simulink model "HvacAirTEstimdAvg"  (R2022b)
settings: Solver=FixedStepDiscrete  StartTime=0.0  StopTime=10.0  FixedStep=0.2  RelTol=1e-3
settings Model: Creator=blue.ge  LastModifiedBy=blue.ge
settings DataIOCC: SaveFormat=StructureWithTime
settings OptimizationCC: DefaultParameterBehavior=Inlined  EnhancedBackFolding=on  PassReuseOutputArgsAs=Individual arguments  ZeroExternalMemoryAtStartup=off  ZeroInternalMemoryAtStartup=off  OptimizeBlockOrder=speed  DifferentSizesBufferReuse=on  OptimizationCustomize=off  EfficientTunableParamExpr=on
settings DebuggingCC: ParameterTunabilityLossMsg=error
settings ModelReferenceCC: ModelReferenceNumInstancesAllowed=Single
settings SFSimCC: MATLABDynamicMemAlloc=off
settings RTWCC: Description=AUTOSAR  SystemTargetFile=autosar.tlc  GenCodeOnly=on  TemplateMakefile=ert_default_tmf
settings CodeAppCC: ForceParamTrailComments=on  IgnoreCustomStorageClasses=off  OperatorAnnotations=on  SimulinkDataObjDesc=on  SFDataObjDesc=on  InsertBlockDesc=on
settings STFCustomTargetCC: TargetLangStandard=C99 (ISO)  MultiwordLength=256  ParMdlRefBuildCompliant=on  CompOptLevelCompliant=on  IncludeMdlTerminateFcn=off  CombineOutputUpdateFcns=on  SuppressErrorStatus=on  ERTCustomFileBanners=on  SupportNonFinite=off  SupportComplex=off  SupportContinuousTime=off  SupportNonInlinedSFcns=off  RemoveResetFunc=on  MATLABClassNameForMDSCustomization=Simulink.SoftwareTarget.ERTCustomization  AutosarCompliant=on  UseToolchainInfoCompliant=on  LookupTableObjectStructAxisOrder=2,1,3,4,...  LUTObjectStructOrderEvenSpacing=Size,Table,Breakpoints  SystemTargetFile=autosar.tlc
settings ERTTargetCC: TargetLangStandard=C99 (ISO)  ConcurrentExecutionCompliant=off  IncludeMdlTerminateFcn=off  SuppressErrorStatus=on  SupportNonFinite=off  SupportComplex=off  ModelStepFunctionPrototypeControlCompliant=off  CPPClassGenCompliant=off  AutosarCompliant=on  LookupTableObjectStructAxisOrder=2,1,3,4,...  LUTObjectStructOrderEvenSpacing=Size,Table,Breakpoints  InlinedParameterPlacement=NonHierarchical  ERTCustomFileTemplate=example_file_process.tlc

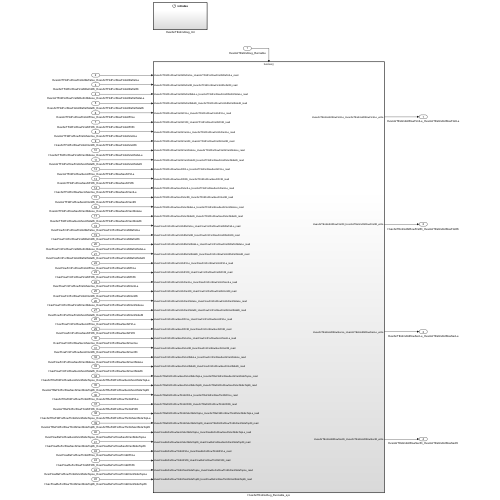
[thumbnail: Simulink's stored preview of the top-level diagram]
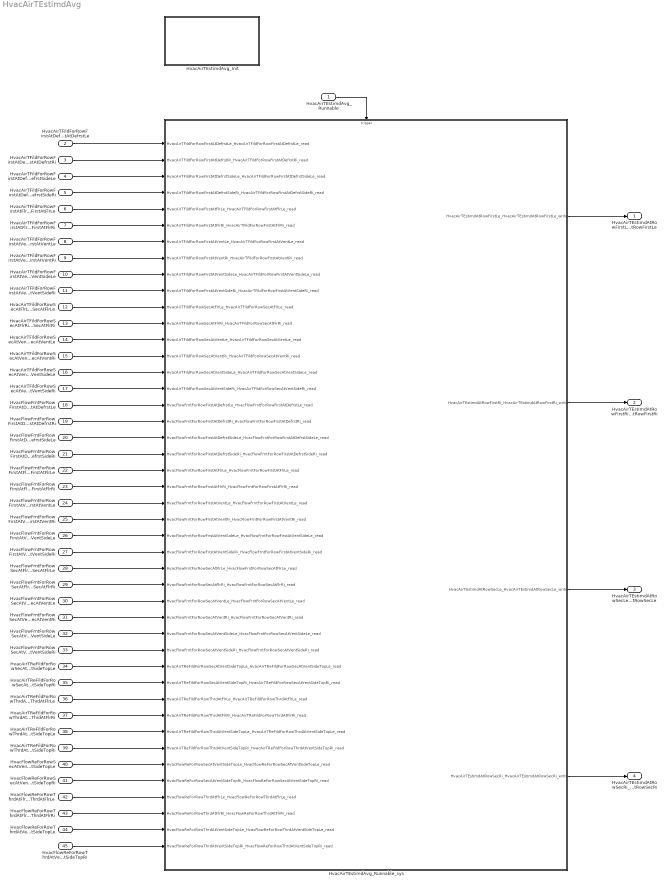
== system HvacAirTEstimdAvg ==
  HvacAirTEstimdAvg_Runnable : Inport  SampleTime=0.2  OutputFunctionCall=on
  HvacAirTFildForRowFirstAtDefrstLe_HvacAirTFildForRowFirstAtDefrstLe : Inport  Port=2  OutDataTypeStr=T30  PortDimensions=1
  HvacAirTFildForRowFirstAtDefrstRi_HvacAirTFildForRowFirstAtDefrstRi : Inport  Port=3  OutDataTypeStr=T30  PortDimensions=1
  HvacAirTFildForRowFirstAtDefrstSideLe_HvacAirTFildForRowFirstAtDefrstSideLe : Inport  Port=4  OutDataTypeStr=T30  PortDimensions=1
  HvacAirTFildForRowFirstAtDefrstSideRi_HvacAirTFildForRowFirstAtDefrstSideRi : Inport  Port=5  OutDataTypeStr=T30  PortDimensions=1
  HvacAirTFildForRowFirstAtFlrLe_HvacAirTFildForRowFirstAtFlrLe : Inport  Port=6  OutDataTypeStr=T30  PortDimensions=1
  HvacAirTFildForRowFirstAtFlrRi_HvacAirTFildForRowFirstAtFlrRi : Inport  Port=7  OutDataTypeStr=T30  PortDimensions=1
  HvacAirTFildForRowFirstAtVentLe_HvacAirTFildForRowFirstAtVentLe : Inport  Port=8  OutDataTypeStr=T30  PortDimensions=1
  HvacAirTFildForRowFirstAtVentRi_HvacAirTFildForRowFirstAtVentRi : Inport  Port=9  OutDataTypeStr=T30  PortDimensions=1
  HvacAirTFildForRowFirstAtVentSideLe_HvacAirTFildForRowFirstAtVentSideLe : Inport  Port=10  OutDataTypeStr=T30  PortDimensions=1
  HvacAirTFildForRowFirstAtVentSideRi_HvacAirTFildForRowFirstAtVentSideRi : Inport  Port=11  OutDataTypeStr=T30  PortDimensions=1
  HvacAirTFildForRowSecAtFlrLe_HvacAirTFildForRowSecAtFlrLe : Inport  Port=12  OutDataTypeStr=T30  PortDimensions=1
  HvacAirTFildForRowSecAtFlrRi_HvacAirTFildForRowSecAtFlrRi : Inport  Port=13  OutDataTypeStr=T30  PortDimensions=1
  HvacAirTFildForRowSecAtVentLe_HvacAirTFildForRowSecAtVentLe : Inport  Port=14  OutDataTypeStr=T30  PortDimensions=1
  HvacAirTFildForRowSecAtVentRi_HvacAirTFildForRowSecAtVentRi : Inport  Port=15  OutDataTypeStr=T30  PortDimensions=1
  HvacAirTFildForRowSecAtVentSideLe_HvacAirTFildForRowSecAtVentSideLe : Inport  Port=16  OutDataTypeStr=T30  PortDimensions=1
  HvacAirTFildForRowSecAtVentSideRi_HvacAirTFildForRowSecAtVentSideRi : Inport  Port=17  OutDataTypeStr=T30  PortDimensions=1
  HvacFlowFrntForRowFirstAtDefrstLe_HvacFlowFrntForRowFirstAtDefrstLe : Inport  Port=18  OutDataTypeStr=AirFlow30  PortDimensions=1
  HvacFlowFrntForRowFirstAtDefrstRi_HvacFlowFrntForRowFirstAtDefrstRi : Inport  Port=19  OutDataTypeStr=AirFlow30  PortDimensions=1
  HvacFlowFrntForRowFirstAtDefrstSideLe_HvacFlowFrntForRowFirstAtDefrstSideLe : Inport  Port=20  OutDataTypeStr=AirFlow30  PortDimensions=1
  HvacFlowFrntForRowFirstAtDefrstSideRi_HvacFlowFrntForRowFirstAtDefrstSideRi : Inport  Port=21  OutDataTypeStr=AirFlow30  PortDimensions=1
  HvacFlowFrntForRowFirstAtFlrLe_HvacFlowFrntForRowFirstAtFlrLe : Inport  Port=22  OutDataTypeStr=AirFlow30  PortDimensions=1
  HvacFlowFrntForRowFirstAtFlrRi_HvacFlowFrntForRowFirstAtFlrRi : Inport  Port=23  OutDataTypeStr=AirFlow30  PortDimensions=1
  HvacFlowFrntForRowFirstAtVentLe_HvacFlowFrntForRowFirstAtVentLe : Inport  Port=24  OutDataTypeStr=AirFlow30  PortDimensions=1
  HvacFlowFrntForRowFirstAtVentRi_HvacFlowFrntForRowFirstAtVentRi : Inport  Port=25  OutDataTypeStr=AirFlow30  PortDimensions=1
  HvacFlowFrntForRowFirstAtVentSideLe_HvacFlowFrntForRowFirstAtVentSideLe : Inport  Port=26  OutDataTypeStr=AirFlow30  PortDimensions=1
  HvacFlowFrntForRowFirstAtVentSideRi_HvacFlowFrntForRowFirstAtVentSideRi : Inport  Port=27  OutDataTypeStr=AirFlow30  PortDimensions=1
  HvacFlowFrntForRowSecAtFlrLe_HvacFlowFrntForRowSecAtFlrLe : Inport  Port=28  OutDataTypeStr=AirFlow30  PortDimensions=1
  HvacFlowFrntForRowSecAtFlrRi_HvacFlowFrntForRowSecAtFlrRi : Inport  Port=29  OutDataTypeStr=AirFlow30  PortDimensions=1
  HvacFlowFrntForRowSecAtVentLe_HvacFlowFrntForRowSecAtVentLe : Inport  Port=30  OutDataTypeStr=AirFlow30  PortDimensions=1
  HvacFlowFrntForRowSecAtVentRi_HvacFlowFrntForRowSecAtVentRi : Inport  Port=31  OutDataTypeStr=AirFlow30  PortDimensions=1
  HvacFlowFrntForRowSecAtVentSideLe_HvacFlowFrntForRowSecAtVentSideLe : Inport  Port=32  OutDataTypeStr=AirFlow30  PortDimensions=1
  HvacFlowFrntForRowSecAtVentSideRi_HvacFlowFrntForRowSecAtVentSideRi : Inport  Port=33  OutDataTypeStr=AirFlow30  PortDimensions=1
  HvacAirTReFildForRowSecAtVentSideTopLe_HvacAirTReFildForRowSecAtVentSideTopLe : Inport  Port=34  OutDataTypeStr=T30  PortDimensions=1
  HvacAirTReFildForRowSecAtVentSideTopRi_HvacAirTReFildForRowSecAtVentSideTopRi : Inport  Port=35  OutDataTypeStr=T30  PortDimensions=1
  HvacAirTReFildForRowThrdAtFlrLe_HvacAirTReFildForRowThrdAtFlrLe : Inport  Port=36  OutDataTypeStr=T30  PortDimensions=1
  HvacAirTReFildForRowThrdAtFlrRi_HvacAirTReFildForRowThrdAtFlrRi : Inport  Port=37  OutDataTypeStr=T30  PortDimensions=1
  HvacAirTReFildForRowThrdAtVentSideTopLe_HvacAirTReFildForRowThrdAtVentSideTopLe : Inport  Port=38  OutDataTypeStr=T30  PortDimensions=1
  HvacAirTReFildForRowThrdAtVentSideTopRi_HvacAirTReFildForRowThrdAtVentSideTopRi : Inport  Port=39  OutDataTypeStr=T30  PortDimensions=1
  HvacFlowReForRowSecAtVentSideTopLe_HvacFlowReForRowSecAtVentSideTopLe : Inport  Port=40  OutDataTypeStr=AirFlow30  PortDimensions=1
  HvacFlowReForRowSecAtVentSideTopRi_HvacFlowReForRowSecAtVentSideTopRi : Inport  Port=41  OutDataTypeStr=AirFlow30  PortDimensions=1
  HvacFlowReForRowThrdAtFlrLe_HvacFlowReForRowThrdAtFlrLe : Inport  Port=42  OutDataTypeStr=AirFlow30  PortDimensions=1
  HvacFlowReForRowThrdAtFlrRi_HvacFlowReForRowThrdAtFlrRi : Inport  Port=43  OutDataTypeStr=AirFlow30  PortDimensions=1
  HvacFlowReForRowThrdAtVentSideTopLe_HvacFlowReForRowThrdAtVentSideTopLe : Inport  Port=44  OutDataTypeStr=AirFlow30  PortDimensions=1
  HvacFlowReForRowThrdAtVentSideTopRi_HvacFlowReForRowThrdAtVentSideTopRi : Inport  Port=45  OutDataTypeStr=AirFlow30  PortDimensions=1
  HvacAirTEstimdAvg_Init : Subsystem
  HvacAirTEstimdAvg_Runnable_sys : Subsystem
  HvacAirTEstimdAtRowFirstLe_HvacAirTEstimdAtRowFirstLe : Outport  OutDataTypeStr=T30  PortDimensions=1
  HvacAirTEstimdAtRowFirstRi_HvacAirTEstimdAtRowFirstRi : Outport  Port=2  OutDataTypeStr=T30  PortDimensions=1
  HvacAirTEstimdAtRowSecLe_HvacAirTEstimdAtRowSecLe : Outport  Port=3  OutDataTypeStr=T30  PortDimensions=1
  HvacAirTEstimdAtRowSecRi_HvacAirTEstimdAtRowSecRi : Outport  Port=4  OutDataTypeStr=T30  PortDimensions=1
  wires:
  HvacAirTFildForRowFirstAtDefrstLe_HvacAirTFildForRowFirstAtDefrstLe/1 -> HvacAirTEstimdAvg_Runnable_sys/1(HvacAirTFildForRowFirstAtDefrstLe_HvacAirTFildForRowFirstAtDefrstLe_read)
  HvacAirTFildForRowFirstAtDefrstRi_HvacAirTFildForRowFirstAtDefrstRi/1 -> HvacAirTEstimdAvg_Runnable_sys/2(HvacAirTFildForRowFirstAtDefrstRi_HvacAirTFildForRowFirstAtDefrstRi_read)
  HvacAirTFildForRowFirstAtDefrstSideLe_HvacAirTFildForRowFirstAtDefrstSideLe/1 -> HvacAirTEstimdAvg_Runnable_sys/3(HvacAirTFildForRowFirstAtDefrstSideLe_HvacAirTFildForRowFirstAtDefrstSideLe_read)
  HvacAirTFildForRowFirstAtDefrstSideRi_HvacAirTFildForRowFirstAtDefrstSideRi/1 -> HvacAirTEstimdAvg_Runnable_sys/4(HvacAirTFildForRowFirstAtDefrstSideRi_HvacAirTFildForRowFirstAtDefrstSideRi_read)
  HvacAirTFildForRowFirstAtFlrLe_HvacAirTFildForRowFirstAtFlrLe/1 -> HvacAirTEstimdAvg_Runnable_sys/5(HvacAirTFildForRowFirstAtFlrLe_HvacAirTFildForRowFirstAtFlrLe_read)
  HvacAirTFildForRowFirstAtFlrRi_HvacAirTFildForRowFirstAtFlrRi/1 -> HvacAirTEstimdAvg_Runnable_sys/6(HvacAirTFildForRowFirstAtFlrRi_HvacAirTFildForRowFirstAtFlrRi_read)
  HvacAirTFildForRowFirstAtVentLe_HvacAirTFildForRowFirstAtVentLe/1 -> HvacAirTEstimdAvg_Runnable_sys/7(HvacAirTFildForRowFirstAtVentLe_HvacAirTFildForRowFirstAtVentLe_read)
  HvacAirTFildForRowFirstAtVentRi_HvacAirTFildForRowFirstAtVentRi/1 -> HvacAirTEstimdAvg_Runnable_sys/8(HvacAirTFildForRowFirstAtVentRi_HvacAirTFildForRowFirstAtVentRi_read)
  HvacAirTFildForRowFirstAtVentSideLe_HvacAirTFildForRowFirstAtVentSideLe/1 -> HvacAirTEstimdAvg_Runnable_sys/9(HvacAirTFildForRowFirstAtVentSideLe_HvacAirTFildForRowFirstAtVentSideLe_read)
  HvacAirTFildForRowFirstAtVentSideRi_HvacAirTFildForRowFirstAtVentSideRi/1 -> HvacAirTEstimdAvg_Runnable_sys/10(HvacAirTFildForRowFirstAtVentSideRi_HvacAirTFildForRowFirstAtVentSideRi_read)
  HvacAirTFildForRowSecAtFlrLe_HvacAirTFildForRowSecAtFlrLe/1 -> HvacAirTEstimdAvg_Runnable_sys/11(HvacAirTFildForRowSecAtFlrLe_HvacAirTFildForRowSecAtFlrLe_read)
  HvacAirTFildForRowSecAtFlrRi_HvacAirTFildForRowSecAtFlrRi/1 -> HvacAirTEstimdAvg_Runnable_sys/12(HvacAirTFildForRowSecAtFlrRi_HvacAirTFildForRowSecAtFlrRi_read)
  HvacAirTFildForRowSecAtVentLe_HvacAirTFildForRowSecAtVentLe/1 -> HvacAirTEstimdAvg_Runnable_sys/13(HvacAirTFildForRowSecAtVentLe_HvacAirTFildForRowSecAtVentLe_read)
  HvacAirTFildForRowSecAtVentRi_HvacAirTFildForRowSecAtVentRi/1 -> HvacAirTEstimdAvg_Runnable_sys/14(HvacAirTFildForRowSecAtVentRi_HvacAirTFildForRowSecAtVentRi_read)
  HvacAirTFildForRowSecAtVentSideLe_HvacAirTFildForRowSecAtVentSideLe/1 -> HvacAirTEstimdAvg_Runnable_sys/15(HvacAirTFildForRowSecAtVentSideLe_HvacAirTFildForRowSecAtVentSideLe_read)
  HvacAirTFildForRowSecAtVentSideRi_HvacAirTFildForRowSecAtVentSideRi/1 -> HvacAirTEstimdAvg_Runnable_sys/16(HvacAirTFildForRowSecAtVentSideRi_HvacAirTFildForRowSecAtVentSideRi_read)
  HvacFlowFrntForRowFirstAtDefrstLe_HvacFlowFrntForRowFirstAtDefrstLe/1 -> HvacAirTEstimdAvg_Runnable_sys/17(HvacFlowFrntForRowFirstAtDefrstLe_HvacFlowFrntForRowFirstAtDefrstLe_read)
  HvacFlowFrntForRowFirstAtDefrstRi_HvacFlowFrntForRowFirstAtDefrstRi/1 -> HvacAirTEstimdAvg_Runnable_sys/18(HvacFlowFrntForRowFirstAtDefrstRi_HvacFlowFrntForRowFirstAtDefrstRi_read)
  HvacFlowFrntForRowFirstAtDefrstSideLe_HvacFlowFrntForRowFirstAtDefrstSideLe/1 -> HvacAirTEstimdAvg_Runnable_sys/19(HvacFlowFrntForRowFirstAtDefrstSideLe_HvacFlowFrntForRowFirstAtDefrstSideLe_read)
  HvacFlowFrntForRowFirstAtDefrstSideRi_HvacFlowFrntForRowFirstAtDefrstSideRi/1 -> HvacAirTEstimdAvg_Runnable_sys/20(HvacFlowFrntForRowFirstAtDefrstSideRi_HvacFlowFrntForRowFirstAtDefrstSideRi_read)
  HvacFlowFrntForRowFirstAtFlrLe_HvacFlowFrntForRowFirstAtFlrLe/1 -> HvacAirTEstimdAvg_Runnable_sys/21(HvacFlowFrntForRowFirstAtFlrLe_HvacFlowFrntForRowFirstAtFlrLe_read)
  HvacFlowFrntForRowFirstAtFlrRi_HvacFlowFrntForRowFirstAtFlrRi/1 -> HvacAirTEstimdAvg_Runnable_sys/22(HvacFlowFrntForRowFirstAtFlrRi_HvacFlowFrntForRowFirstAtFlrRi_read)
  HvacFlowFrntForRowFirstAtVentLe_HvacFlowFrntForRowFirstAtVentLe/1 -> HvacAirTEstimdAvg_Runnable_sys/23(HvacFlowFrntForRowFirstAtVentLe_HvacFlowFrntForRowFirstAtVentLe_read)
  HvacFlowFrntForRowFirstAtVentRi_HvacFlowFrntForRowFirstAtVentRi/1 -> HvacAirTEstimdAvg_Runnable_sys/24(HvacFlowFrntForRowFirstAtVentRi_HvacFlowFrntForRowFirstAtVentRi_read)
  HvacFlowFrntForRowFirstAtVentSideLe_HvacFlowFrntForRowFirstAtVentSideLe/1 -> HvacAirTEstimdAvg_Runnable_sys/25(HvacFlowFrntForRowFirstAtVentSideLe_HvacFlowFrntForRowFirstAtVentSideLe_read)
  HvacFlowFrntForRowFirstAtVentSideRi_HvacFlowFrntForRowFirstAtVentSideRi/1 -> HvacAirTEstimdAvg_Runnable_sys/26(HvacFlowFrntForRowFirstAtVentSideRi_HvacFlowFrntForRowFirstAtVentSideRi_read)
  HvacFlowFrntForRowSecAtFlrLe_HvacFlowFrntForRowSecAtFlrLe/1 -> HvacAirTEstimdAvg_Runnable_sys/27(HvacFlowFrntForRowSecAtFlrLe_HvacFlowFrntForRowSecAtFlrLe_read)
  HvacFlowFrntForRowSecAtFlrRi_HvacFlowFrntForRowSecAtFlrRi/1 -> HvacAirTEstimdAvg_Runnable_sys/28(HvacFlowFrntForRowSecAtFlrRi_HvacFlowFrntForRowSecAtFlrRi_read)
  HvacFlowFrntForRowSecAtVentLe_HvacFlowFrntForRowSecAtVentLe/1 -> HvacAirTEstimdAvg_Runnable_sys/29(HvacFlowFrntForRowSecAtVentLe_HvacFlowFrntForRowSecAtVentLe_read)
  HvacFlowFrntForRowSecAtVentRi_HvacFlowFrntForRowSecAtVentRi/1 -> HvacAirTEstimdAvg_Runnable_sys/30(HvacFlowFrntForRowSecAtVentRi_HvacFlowFrntForRowSecAtVentRi_read)
  HvacFlowFrntForRowSecAtVentSideLe_HvacFlowFrntForRowSecAtVentSideLe/1 -> HvacAirTEstimdAvg_Runnable_sys/31(HvacFlowFrntForRowSecAtVentSideLe_HvacFlowFrntForRowSecAtVentSideLe_read)
  HvacFlowFrntForRowSecAtVentSideRi_HvacFlowFrntForRowSecAtVentSideRi/1 -> HvacAirTEstimdAvg_Runnable_sys/32(HvacFlowFrntForRowSecAtVentSideRi_HvacFlowFrntForRowSecAtVentSideRi_read)
  HvacAirTReFildForRowSecAtVentSideTopLe_HvacAirTReFildForRowSecAtVentSideTopLe/1 -> HvacAirTEstimdAvg_Runnable_sys/33(HvacAirTReFildForRowSecAtVentSideTopLe_HvacAirTReFildForRowSecAtVentSideTopLe_read)
  HvacAirTReFildForRowSecAtVentSideTopRi_HvacAirTReFildForRowSecAtVentSideTopRi/1 -> HvacAirTEstimdAvg_Runnable_sys/34(HvacAirTReFildForRowSecAtVentSideTopRi_HvacAirTReFildForRowSecAtVentSideTopRi_read)
  HvacAirTReFildForRowThrdAtFlrLe_HvacAirTReFildForRowThrdAtFlrLe/1 -> HvacAirTEstimdAvg_Runnable_sys/35(HvacAirTReFildForRowThrdAtFlrLe_HvacAirTReFildForRowThrdAtFlrLe_read)
  HvacAirTReFildForRowThrdAtFlrRi_HvacAirTReFildForRowThrdAtFlrRi/1 -> HvacAirTEstimdAvg_Runnable_sys/36(HvacAirTReFildForRowThrdAtFlrRi_HvacAirTReFildForRowThrdAtFlrRi_read)
  HvacAirTReFildForRowThrdAtVentSideTopLe_HvacAirTReFildForRowThrdAtVentSideTopLe/1 -> HvacAirTEstimdAvg_Runnable_sys/37(HvacAirTReFildForRowThrdAtVentSideTopLe_HvacAirTReFildForRowThrdAtVentSideTopLe_read)
  HvacAirTReFildForRowThrdAtVentSideTopRi_HvacAirTReFildForRowThrdAtVentSideTopRi/1 -> HvacAirTEstimdAvg_Runnable_sys/38(HvacAirTReFildForRowThrdAtVentSideTopRi_HvacAirTReFildForRowThrdAtVentSideTopRi_read)
  HvacFlowReForRowSecAtVentSideTopLe_HvacFlowReForRowSecAtVentSideTopLe/1 -> HvacAirTEstimdAvg_Runnable_sys/39(HvacFlowReForRowSecAtVentSideTopLe_HvacFlowReForRowSecAtVentSideTopLe_read)
  HvacFlowReForRowSecAtVentSideTopRi_HvacFlowReForRowSecAtVentSideTopRi/1 -> HvacAirTEstimdAvg_Runnable_sys/40(HvacFlowReForRowSecAtVentSideTopRi_HvacFlowReForRowSecAtVentSideTopRi_read)
  HvacFlowReForRowThrdAtFlrLe_HvacFlowReForRowThrdAtFlrLe/1 -> HvacAirTEstimdAvg_Runnable_sys/41(HvacFlowReForRowThrdAtFlrLe_HvacFlowReForRowThrdAtFlrLe_read)
  HvacFlowReForRowThrdAtFlrRi_HvacFlowReForRowThrdAtFlrRi/1 -> HvacAirTEstimdAvg_Runnable_sys/42(HvacFlowReForRowThrdAtFlrRi_HvacFlowReForRowThrdAtFlrRi_read)
  HvacFlowReForRowThrdAtVentSideTopLe_HvacFlowReForRowThrdAtVentSideTopLe/1 -> HvacAirTEstimdAvg_Runnable_sys/43(HvacFlowReForRowThrdAtVentSideTopLe_HvacFlowReForRowThrdAtVentSideTopLe_read)
  HvacFlowReForRowThrdAtVentSideTopRi_HvacFlowReForRowThrdAtVentSideTopRi/1 -> HvacAirTEstimdAvg_Runnable_sys/44(HvacFlowReForRowThrdAtVentSideTopRi_HvacFlowReForRowThrdAtVentSideTopRi_read)
  HvacAirTEstimdAvg_Runnable_sys/1(HvacAirTEstimdAtRowFirstLe_HvacAirTEstimdAtRowFirstLe_write) -> HvacAirTEstimdAtRowFirstLe_HvacAirTEstimdAtRowFirstLe/1
  HvacAirTEstimdAvg_Runnable_sys/2(HvacAirTEstimdAtRowFirstRi_HvacAirTEstimdAtRowFirstRi_write) -> HvacAirTEstimdAtRowFirstRi_HvacAirTEstimdAtRowFirstRi/1
  HvacAirTEstimdAvg_Runnable_sys/3(HvacAirTEstimdAtRowSecLe_HvacAirTEstimdAtRowSecLe_write) -> HvacAirTEstimdAtRowSecLe_HvacAirTEstimdAtRowSecLe/1
  HvacAirTEstimdAvg_Runnable_sys/4(HvacAirTEstimdAtRowSecRi_HvacAirTEstimdAtRowSecRi_write) -> HvacAirTEstimdAtRowSecRi_HvacAirTEstimdAtRowSecRi/1
  HvacAirTEstimdAvg_Runnable/1 -> HvacAirTEstimdAvg_Runnable_sys/Trigger

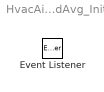
== system HvacAirTEstimdAvg/HvacAirTEstimdAvg_Init ==
  Event Listener : EventListener  EventName=reset

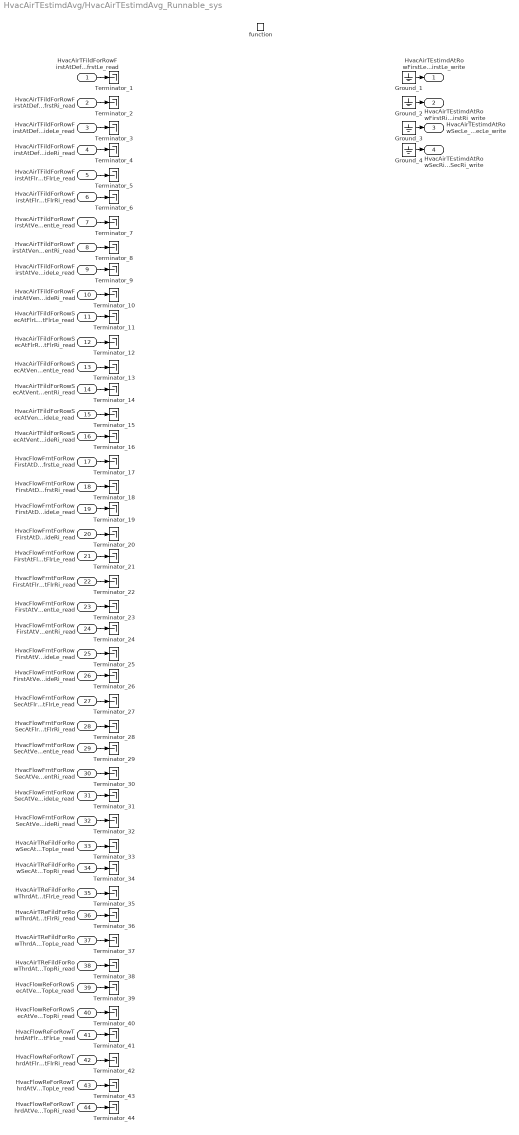
== system HvacAirTEstimdAvg/HvacAirTEstimdAvg_Runnable_sys ==
  HvacAirTFildForRowFirstAtDefrstLe_HvacAirTFildForRowFirstAtDefrstLe_read : Inport
  HvacAirTFildForRowFirstAtDefrstRi_HvacAirTFildForRowFirstAtDefrstRi_read : Inport  Port=2
  HvacAirTFildForRowFirstAtDefrstSideLe_HvacAirTFildForRowFirstAtDefrstSideLe_read : Inport  Port=3
  HvacAirTFildForRowFirstAtDefrstSideRi_HvacAirTFildForRowFirstAtDefrstSideRi_read : Inport  Port=4
  HvacAirTFildForRowFirstAtFlrLe_HvacAirTFildForRowFirstAtFlrLe_read : Inport  Port=5
  HvacAirTFildForRowFirstAtFlrRi_HvacAirTFildForRowFirstAtFlrRi_read : Inport  Port=6
  HvacAirTFildForRowFirstAtVentLe_HvacAirTFildForRowFirstAtVentLe_read : Inport  Port=7
  HvacAirTFildForRowFirstAtVentRi_HvacAirTFildForRowFirstAtVentRi_read : Inport  Port=8
  HvacAirTFildForRowFirstAtVentSideLe_HvacAirTFildForRowFirstAtVentSideLe_read : Inport  Port=9
  HvacAirTFildForRowFirstAtVentSideRi_HvacAirTFildForRowFirstAtVentSideRi_read : Inport  Port=10
  HvacAirTFildForRowSecAtFlrLe_HvacAirTFildForRowSecAtFlrLe_read : Inport  Port=11
  HvacAirTFildForRowSecAtFlrRi_HvacAirTFildForRowSecAtFlrRi_read : Inport  Port=12
  HvacAirTFildForRowSecAtVentLe_HvacAirTFildForRowSecAtVentLe_read : Inport  Port=13
  HvacAirTFildForRowSecAtVentRi_HvacAirTFildForRowSecAtVentRi_read : Inport  Port=14
  HvacAirTFildForRowSecAtVentSideLe_HvacAirTFildForRowSecAtVentSideLe_read : Inport  Port=15
  HvacAirTFildForRowSecAtVentSideRi_HvacAirTFildForRowSecAtVentSideRi_read : Inport  Port=16
  HvacFlowFrntForRowFirstAtDefrstLe_HvacFlowFrntForRowFirstAtDefrstLe_read : Inport  Port=17
  HvacFlowFrntForRowFirstAtDefrstRi_HvacFlowFrntForRowFirstAtDefrstRi_read : Inport  Port=18
  HvacFlowFrntForRowFirstAtDefrstSideLe_HvacFlowFrntForRowFirstAtDefrstSideLe_read : Inport  Port=19
  HvacFlowFrntForRowFirstAtDefrstSideRi_HvacFlowFrntForRowFirstAtDefrstSideRi_read : Inport  Port=20
  HvacFlowFrntForRowFirstAtFlrLe_HvacFlowFrntForRowFirstAtFlrLe_read : Inport  Port=21
  HvacFlowFrntForRowFirstAtFlrRi_HvacFlowFrntForRowFirstAtFlrRi_read : Inport  Port=22
  HvacFlowFrntForRowFirstAtVentLe_HvacFlowFrntForRowFirstAtVentLe_read : Inport  Port=23
  HvacFlowFrntForRowFirstAtVentRi_HvacFlowFrntForRowFirstAtVentRi_read : Inport  Port=24
  HvacFlowFrntForRowFirstAtVentSideLe_HvacFlowFrntForRowFirstAtVentSideLe_read : Inport  Port=25
  HvacFlowFrntForRowFirstAtVentSideRi_HvacFlowFrntForRowFirstAtVentSideRi_read : Inport  Port=26
  HvacFlowFrntForRowSecAtFlrLe_HvacFlowFrntForRowSecAtFlrLe_read : Inport  Port=27
  HvacFlowFrntForRowSecAtFlrRi_HvacFlowFrntForRowSecAtFlrRi_read : Inport  Port=28
  HvacFlowFrntForRowSecAtVentLe_HvacFlowFrntForRowSecAtVentLe_read : Inport  Port=29
  HvacFlowFrntForRowSecAtVentRi_HvacFlowFrntForRowSecAtVentRi_read : Inport  Port=30
  HvacFlowFrntForRowSecAtVentSideLe_HvacFlowFrntForRowSecAtVentSideLe_read : Inport  Port=31
  HvacFlowFrntForRowSecAtVentSideRi_HvacFlowFrntForRowSecAtVentSideRi_read : Inport  Port=32
  HvacAirTReFildForRowSecAtVentSideTopLe_HvacAirTReFildForRowSecAtVentSideTopLe_read : Inport  Port=33
  HvacAirTReFildForRowSecAtVentSideTopRi_HvacAirTReFildForRowSecAtVentSideTopRi_read : Inport  Port=34
  HvacAirTReFildForRowThrdAtFlrLe_HvacAirTReFildForRowThrdAtFlrLe_read : Inport  Port=35
  HvacAirTReFildForRowThrdAtFlrRi_HvacAirTReFildForRowThrdAtFlrRi_read : Inport  Port=36
  HvacAirTReFildForRowThrdAtVentSideTopLe_HvacAirTReFildForRowThrdAtVentSideTopLe_read : Inport  Port=37
  HvacAirTReFildForRowThrdAtVentSideTopRi_HvacAirTReFildForRowThrdAtVentSideTopRi_read : Inport  Port=38
  HvacFlowReForRowSecAtVentSideTopLe_HvacFlowReForRowSecAtVentSideTopLe_read : Inport  Port=39
  HvacFlowReForRowSecAtVentSideTopRi_HvacFlowReForRowSecAtVentSideTopRi_read : Inport  Port=40
  HvacFlowReForRowThrdAtFlrLe_HvacFlowReForRowThrdAtFlrLe_read : Inport  Port=41
  HvacFlowReForRowThrdAtFlrRi_HvacFlowReForRowThrdAtFlrRi_read : Inport  Port=42
  HvacFlowReForRowThrdAtVentSideTopLe_HvacFlowReForRowThrdAtVentSideTopLe_read : Inport  Port=43
  HvacFlowReForRowThrdAtVentSideTopRi_HvacFlowReForRowThrdAtVentSideTopRi_read : Inport  Port=44
  function : TriggerPort  TriggerType=function-call  VariantControl=(inherit)  SampleTimeType=periodic  SampleTime=-1
  Ground_{1..4} (4 alike) : Ground
  Terminator_{1..44} (44 alike) : Terminator
  HvacAirTEstimdAtRowFirstLe_HvacAirTEstimdAtRowFirstLe_write : Outport
      settings: EnsureOutportIsVirtual=on
  HvacAirTEstimdAtRowFirstRi_HvacAirTEstimdAtRowFirstRi_write : Outport  Port=2
      settings: EnsureOutportIsVirtual=on
  HvacAirTEstimdAtRowSecLe_HvacAirTEstimdAtRowSecLe_write : Outport  Port=3
      settings: EnsureOutportIsVirtual=on
  HvacAirTEstimdAtRowSecRi_HvacAirTEstimdAtRowSecRi_write : Outport  Port=4
      settings: EnsureOutportIsVirtual=on
  wires:
  HvacAirTFildForRowFirstAtDefrstLe_HvacAirTFildForRowFirstAtDefrstLe_read/1 -> Terminator_1/1
  HvacAirTFildForRowFirstAtDefrstRi_HvacAirTFildForRowFirstAtDefrstRi_read/1 -> Terminator_2/1
  HvacAirTFildForRowFirstAtDefrstSideLe_HvacAirTFildForRowFirstAtDefrstSideLe_read/1 -> Terminator_3/1
  HvacAirTFildForRowFirstAtDefrstSideRi_HvacAirTFildForRowFirstAtDefrstSideRi_read/1 -> Terminator_4/1
  HvacAirTFildForRowFirstAtFlrLe_HvacAirTFildForRowFirstAtFlrLe_read/1 -> Terminator_5/1
  HvacAirTFildForRowFirstAtFlrRi_HvacAirTFildForRowFirstAtFlrRi_read/1 -> Terminator_6/1
  HvacAirTFildForRowFirstAtVentLe_HvacAirTFildForRowFirstAtVentLe_read/1 -> Terminator_7/1
  HvacAirTFildForRowFirstAtVentRi_HvacAirTFildForRowFirstAtVentRi_read/1 -> Terminator_8/1
  HvacAirTFildForRowFirstAtVentSideLe_HvacAirTFildForRowFirstAtVentSideLe_read/1 -> Terminator_9/1
  HvacAirTFildForRowFirstAtVentSideRi_HvacAirTFildForRowFirstAtVentSideRi_read/1 -> Terminator_10/1
  HvacAirTFildForRowSecAtFlrLe_HvacAirTFildForRowSecAtFlrLe_read/1 -> Terminator_11/1
  HvacAirTFildForRowSecAtFlrRi_HvacAirTFildForRowSecAtFlrRi_read/1 -> Terminator_12/1
  HvacAirTFildForRowSecAtVentLe_HvacAirTFildForRowSecAtVentLe_read/1 -> Terminator_13/1
  HvacAirTFildForRowSecAtVentRi_HvacAirTFildForRowSecAtVentRi_read/1 -> Terminator_14/1
  HvacAirTFildForRowSecAtVentSideLe_HvacAirTFildForRowSecAtVentSideLe_read/1 -> Terminator_15/1
  HvacAirTFildForRowSecAtVentSideRi_HvacAirTFildForRowSecAtVentSideRi_read/1 -> Terminator_16/1
  HvacFlowFrntForRowFirstAtDefrstLe_HvacFlowFrntForRowFirstAtDefrstLe_read/1 -> Terminator_17/1
  HvacFlowFrntForRowFirstAtDefrstRi_HvacFlowFrntForRowFirstAtDefrstRi_read/1 -> Terminator_18/1
  HvacFlowFrntForRowFirstAtDefrstSideLe_HvacFlowFrntForRowFirstAtDefrstSideLe_read/1 -> Terminator_19/1
  HvacFlowFrntForRowFirstAtDefrstSideRi_HvacFlowFrntForRowFirstAtDefrstSideRi_read/1 -> Terminator_20/1
  HvacFlowFrntForRowFirstAtFlrLe_HvacFlowFrntForRowFirstAtFlrLe_read/1 -> Terminator_21/1
  HvacFlowFrntForRowFirstAtFlrRi_HvacFlowFrntForRowFirstAtFlrRi_read/1 -> Terminator_22/1
  HvacFlowFrntForRowFirstAtVentLe_HvacFlowFrntForRowFirstAtVentLe_read/1 -> Terminator_23/1
  HvacFlowFrntForRowFirstAtVentRi_HvacFlowFrntForRowFirstAtVentRi_read/1 -> Terminator_24/1
  HvacFlowFrntForRowFirstAtVentSideLe_HvacFlowFrntForRowFirstAtVentSideLe_read/1 -> Terminator_25/1
  HvacFlowFrntForRowFirstAtVentSideRi_HvacFlowFrntForRowFirstAtVentSideRi_read/1 -> Terminator_26/1
  HvacFlowFrntForRowSecAtFlrLe_HvacFlowFrntForRowSecAtFlrLe_read/1 -> Terminator_27/1
  HvacFlowFrntForRowSecAtFlrRi_HvacFlowFrntForRowSecAtFlrRi_read/1 -> Terminator_28/1
  HvacFlowFrntForRowSecAtVentLe_HvacFlowFrntForRowSecAtVentLe_read/1 -> Terminator_29/1
  HvacFlowFrntForRowSecAtVentRi_HvacFlowFrntForRowSecAtVentRi_read/1 -> Terminator_30/1
  HvacFlowFrntForRowSecAtVentSideLe_HvacFlowFrntForRowSecAtVentSideLe_read/1 -> Terminator_31/1
  HvacFlowFrntForRowSecAtVentSideRi_HvacFlowFrntForRowSecAtVentSideRi_read/1 -> Terminator_32/1
  HvacAirTReFildForRowSecAtVentSideTopLe_HvacAirTReFildForRowSecAtVentSideTopLe_read/1 -> Terminator_33/1
  HvacAirTReFildForRowSecAtVentSideTopRi_HvacAirTReFildForRowSecAtVentSideTopRi_read/1 -> Terminator_34/1
  HvacAirTReFildForRowThrdAtFlrLe_HvacAirTReFildForRowThrdAtFlrLe_read/1 -> Terminator_35/1
  HvacAirTReFildForRowThrdAtFlrRi_HvacAirTReFildForRowThrdAtFlrRi_read/1 -> Terminator_36/1
  HvacAirTReFildForRowThrdAtVentSideTopLe_HvacAirTReFildForRowThrdAtVentSideTopLe_read/1 -> Terminator_37/1
  HvacAirTReFildForRowThrdAtVentSideTopRi_HvacAirTReFildForRowThrdAtVentSideTopRi_read/1 -> Terminator_38/1
  HvacFlowReForRowSecAtVentSideTopLe_HvacFlowReForRowSecAtVentSideTopLe_read/1 -> Terminator_39/1
  HvacFlowReForRowSecAtVentSideTopRi_HvacFlowReForRowSecAtVentSideTopRi_read/1 -> Terminator_40/1
  HvacFlowReForRowThrdAtFlrLe_HvacFlowReForRowThrdAtFlrLe_read/1 -> Terminator_41/1
  HvacFlowReForRowThrdAtFlrRi_HvacFlowReForRowThrdAtFlrRi_read/1 -> Terminator_42/1
  HvacFlowReForRowThrdAtVentSideTopLe_HvacFlowReForRowThrdAtVentSideTopLe_read/1 -> Terminator_43/1
  HvacFlowReForRowThrdAtVentSideTopRi_HvacFlowReForRowThrdAtVentSideTopRi_read/1 -> Terminator_44/1
  Ground_1/1 -> HvacAirTEstimdAtRowFirstLe_HvacAirTEstimdAtRowFirstLe_write/1
  Ground_2/1 -> HvacAirTEstimdAtRowFirstRi_HvacAirTEstimdAtRowFirstRi_write/1
  Ground_3/1 -> HvacAirTEstimdAtRowSecLe_HvacAirTEstimdAtRowSecLe_write/1
  Ground_4/1 -> HvacAirTEstimdAtRowSecRi_HvacAirTEstimdAtRowSecRi_write/1
